simulink model "test_results_plot"  (R2023b)
settings: Solver=VariableStepAuto  StartTime=0.0  StopTime=800  RelTol=1e-3
model workspace (MAT data in the model file):
  Logfile_Data_Object : robotics.ros.BagSelection (value not decoded)
  Logfile_Path = '/Users/marystirlingbrown/Downloads/2023_11_30_20_10_48_2T3MWRFVXLW056972okayestcontroller.bag'
  Logfile_Playback_Duration = nan
  Logfile_Playback_Offset = nan
libraries: robotlib x4
settings Model: Creator=marystirlingbrown  LastModifiedBy=marystirlingbrown

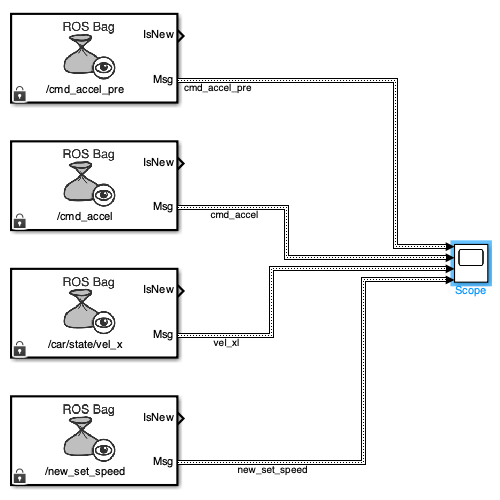
[thumbnail: Simulink's stored preview of the top-level diagram]
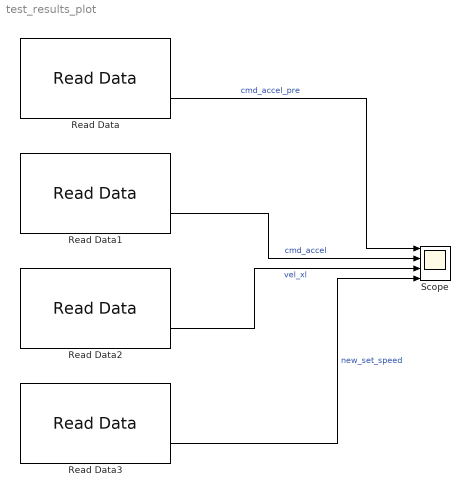
== system test_results_plot ==
  Read Data : robotlib/Read Data  topic=/cmd_accel_pre  sampleTime=-1  logfileType=rosbag  modelName=untitled  msgType=std_msgs/Float64
  Read Data1 : robotlib/Read Data  topic=/cmd_accel  sampleTime=-1  logfileType=rosbag  modelName=untitled  msgType=std_msgs/Float64
  Read Data2 : robotlib/Read Data  topic=/car/state/vel_x  sampleTime=-1  logfileType=rosbag  modelName=untitled  msgType=std_msgs/Float64
  Read Data3 : robotlib/Read Data  topic=/new_set_speed  sampleTime=-1  logfileType=rosbag  modelName=untitled  msgType=std_msgs/Float64
  Scope : Scope  NumInputPorts=4
  wires:
  Read Data/2 -> Scope/1  "cmd_accel_pre"
  Read Data1/2 -> Scope/2  "cmd_accel"
  Read Data2/2 -> Scope/3  "vel_xl"
  Read Data3/2 -> Scope/4  "new_set_speed"
